simulink model "tut3"  (Simulink 7.4)
settings: Solver=ode45  StartTime=0.0  StopTime=10.0  RelTol=1e-3
undefined symbols (not defined in the file): gcbh, pfb_coeff_gen_calc, xlBlockLoadCallback
libraries: xbsIndex_r4 x2537, casper_library_misc x93, casper_library_delays x38, xps_library x10, xbsReplacements_r4 x2, casper_library_pfbs x2, simulink x1, casper_library_ffts_twiddle x1
(part 17 of 44)

== system tut3/fft_wideband_real1/fft_direct/butterfly2_1/twiddle_general_dsp48e ==
  a : Inport
  b : Inport  Port=2
  sync : Inport  Port=3
  a_c_to_ri : casper_library_misc/c_to_ri  SystemSampleTime=-1  n_bits=input_bit_width  bin_pt=input_bit_width-1
  a_delay : xbsIndex_r4/Delay  en=off  latency=bram_latency + 4 + conv_latency  dbl_ovrd=off  reg_retiming=off  block_type=delay
      settings: block_version=11.4
  b_c_to_ri : casper_library_misc/c_to_ri  SystemSampleTime=-1  n_bits=input_bit_width  bin_pt=input_bit_width-1
  b_delay : xbsIndex_r4/Delay  en=off  latency=bram_latency  dbl_ovrd=off  reg_retiming=on  block_type=delay
      settings: block_version=11.4
  cmult : Subsystem  AncestorBlock=casper_library_multipliers/cmult_dsp48e  mask(cmult_dsp48e){n_bits_a=input_bit_width, bin_pt_a=input_bit_width - 1, n_bits_b=coeff_bit_width, bin_pt_b=coeff_bit_width - 2, conjugated=off, full_precision=off, n_bits_c=input_bit_width + 4, bin_pt_c=input_bit_width + 1, quantization=Round  (unbiased: Even Values), overflow=Wrap, cast_latency=conv_latency}
      mask prompts: n_bits_a "Bit width 'a'"; bin_pt_a "Binary point 'a'"; n_bits_b "Bit width 'b'"; bin_pt_b "Binary point 'b'"; conjugated "Conjugate"; full_precision "Full precision"; n_bits_c "Bit width out"; bin_pt_c "Binary point out"; cast_latency "Cast Latency"
      mask help:
      | Multiplies two complex numbers using 4 DSP48Es.
      | Conjugation is optional.
      |
      | Re{a} and Im{a} can be up to 25 bits.
      | Re{b} and Im{b} can be up to 18 bits.
      mask init: same as tut3/fft_wideband_real1/fft_direct/butterfly1_0/twiddle_general_dsp48e/cmult
      settings: AttributesFormatString=|
      | 18_17 * 18_16 ==> 22_19
      | Latency=5
      mask StyleString: edit,edit,edit,edit,checkbox,checkbox,edit,edit,popup(Truncate|Round  (unbiased: +/- Inf)|Round  (unbiased: Even Values)),popup(Wrap|Saturate|Flag as error),edit
      mask CallbackString:
      | |||||full_precision = get_param(gcb, 'full_precision');
      | 
      | if strcmp(full_precision, 'on'),
      |     not_full_precision = 'off';
      | else
      |     not_full_precision = 'on';
      | end
      | 
      | set_param_state(gcb, 'n_bits_c', not_full_precision);
      | set_param_state(gcb, 'bin_pt_c', not_full_precision);
      | set_param_state(gcb, 'quantization', not_full_precision);
      | set_param_state(gcb, 'overflow', not_full_precision);
      | set_param_state(gcb, 'cast_latency', not_full_precision);|||||
      mask TabNameString: Basic,Basic,Basic,Basic,Basic,Output Type,Output Type,Output Type,Output Type,Output Type,Output Type
  coeff_c_to_ri : casper_library_misc/c_to_ri  SystemSampleTime=-1  n_bits=coeff_bit_width  bin_pt=coeff_bit_width-2
      settings: AttributesFormatString=18_16 r/i
  coeff_gen : Subsystem  AncestorBlock=casper_library_ffts_twiddle_coeff_gen/coeff_gen  mask(coeff_gen){Coeffs=[6.123233995736766035868820147292e-17-1i      -0.0015339801862846436358162049984344-0.99999882345170187925020854891045i       -0.003067956762966015608201653108722-0.99999529380957619117964441102231i      -0.0046019261204485496785654419227285-0.99998941108192840321322591989883i      -0.0061358846491543928697298682095607-0.99998117528260110908888691483298i      -0.0076698287395309547656574444829403-0.99997058643097413987987920336309i      -0.0092037547820598367837030551186217-0.99995764455196389786095778617891i       -0.010737659167264448911716101520142-0.99994234967602391161989316969994i       -0.012271538285719823038744102916553-0.99992470183914450299056397852837i       -0.013805388528060225789007020580357-0.99990470108285289807525941796484i       -0.015339206284988098419330704302865-0.99988234745421256111086449891445i        -0.01687298794728165143852471885566-0.99985764100582386060267481298069i       -0.018406729905804695285320704556398-0.99983058179582340319058175737155i       -0.019940428551514476074313364506452-0.99980116988788425569367745993077i       -0.021474080275469483586325836199649-0.99976940535121527897644000404398i       -0.023007681468839288885241955995298-0.99973528826056168306024574121693i       -0.024541228522912142406831037533266-0.99969881869620424996725205346593i       -0.026074717829103914723543056197741-0.99965999674395922269809489080217i       -0.027608145778965698102780379485921-0.99961882249517863829879615877871i       -0.029141508764193618102877891828939-0.99957529604674921763773909333395i       -0.030674803176636459417503388635851-0.99952941750109314256178549840115i       -0.032208025408304578884877145128485-0.99948118696616694567325112075196i       -0.033741171851377517454384502570974-0.99943060455546173237451057502767i        -0.035274238898213822190896848951525-0.9993776703880028478010899561923i       -0.036807222941358866408201322428795-0.99932238458834954375475945198559i       -0.038340120373552666332983562824666-0.99926474728659442359202103034477i       -0.039872927587739727395277355981307-0.99920475861836388631331828946713i       -0.041405640977076593745032084825652-0.99914241872481690531770937013789i        -0.04293825693494083411971828923015-0.99907772775264536146977434327709i       -0.044470771854938619116470732706148-0.99901068585407337696580043484573i       -0.046003182130914518910280719410366-0.99894129318685687124457217578311i       -0.047535484156959136037290392096111-0.99886954991428356098737140200683i       -0.049067674327418007995671445087282-0.99879545620517240500646494183457i       -0.050599749036899212273343806600678-0.99871901223387293811128984089009i       -0.052131704680283191821743571381376-0.99864021818026527110845336210332i       -0.053663537652730554372570992427427-0.99855907422975931364561574810068i       -0.055195244349689913387901896157928-0.99847558057329477421149022120517i       -0.056726821166907658022804383790572-0.99838973740734016093512082079542i       -0.058258264500435606725314841014551-0.99830154493389289260818486582139i       -0.059789570746639882081741035335654-0.99821100336047818846196832964779i       -0.061320736302208529722168606213017-0.99811811290014917918966830256977i       -0.062851757564161309099759478158376-0.99802287377148624081257821671898i         -0.0643826309298572846406827352439-0.99792528619859599547936568342266i       -0.065913352797003804672826277055719-0.99782535041111164453297988075064i        -0.067443919563663981553425230686116-0.9977230666441916362430220033275i       -0.068974327628266607348450634162873-0.99761843513851955478344279981684i       -0.070504573389613897638206196916144-0.99751145614030345409872779782745i       -0.072034653246889290834076291503152-0.99740212990127530279238499133498i       -0.073564563599667329163445117501396-0.99729045667869020697082760307239i        -0.07509430084792116655290072912976-0.99717643673532618819876915949862i       -0.076623861392031505923583267758659-0.99706007033948296225389640312642i ...(+76861 chars)], coeff_bit_width=coeff_bit_width, StepPeriod=0, bram_latency=bram_latency, coeffs_bram=on}
      mask prompts: Coeffs "Coefficients: (0 to 2^(FFTSize-1)-1)"; coeff_bit_width "Coefficient Bit Width:"; StepPeriod "Step Period: (2^?)"; bram_latency "Latency:"; coeffs_bram "Store coefficients in BRAM"
      mask help: Generates a sequence of complex coefficients
      mask init:
      | coeff_gen_init( gcb, ...
      |     'Coeffs', Coeffs, ...
      |     'coeff_bit_width', coeff_bit_width, ...
      |     'StepPeriod', StepPeriod, ...
      |     'bram_latency', bram_latency, ...
      |     'coeffs_bram', coeffs_bram);
      settings: AttributesFormatString=1024 @ (18,16)
      mask CallbackString:
      | Coeffs = eval(get_param(gcb, 'Coeffs'));
      | if( length(Coeffs) == 1 ),
      |     set_param(gcb, 'MaskVisibilities',{'on','on','off','off','off'});
      | else
      |     set_param(gcb, 'MaskVisibilities',{'on','on','on','on','on'});
      | end||||
  sync_delay : xbsIndex_r4/Delay  en=off  latency=bram_latency + 4 + conv_latency  dbl_ovrd=off  reg_retiming=off  block_type=delay
      settings: block_version=11.4
  a_re : Outport
  a_im : Outport  Port=2
  bw_re : Outport  Port=3
  bw_im : Outport  Port=4
  sync_out : Outport  Port=5
  wires:
  a/1 -> a_delay/1
  a_delay/1 -> a_c_to_ri/1
  a_c_to_ri/1 -> a_re/1
  a_c_to_ri/2 -> a_im/1
  b/1 -> b_delay/1
  b_delay/1 -> b_c_to_ri/1
  b_c_to_ri/1 -> cmult/1(a_re)
  b_c_to_ri/2 -> cmult/2(a_im)
  sync/1 -> coeff_gen/1(rst), sync_delay/1
  coeff_gen/1(w) -> coeff_c_to_ri/1
  coeff_c_to_ri/1 -> cmult/3(b_re)
  coeff_c_to_ri/2 -> cmult/4(b_im)
  cmult/1(c_re) -> bw_re/1
  cmult/2(c_im) -> bw_im/1
  sync_delay/1 -> sync_out/1
